simulink model "MX_Gateway"  (Simulink 9.0) (continued)
== stateflow chart MX_Gtwy_Control/System/EMEA Control Mode/Control Modes ==
  state Non_Functional
    en:
    mode_LeftControl = MODE_LEFT_OFFSET;
    mode_RightControl = MODE_RIGHT_AUX1;
    check_LeftNeutral = 0;
    check_RightNeutral = 0;
    flag_AuxEnableFlash = 0;
    aux_inhibit = 0;
    display_request = 0;
    stored_ControlMode = EE_NA_NA;
  state OptionA
    state Left_Control_or_Diverter_Fault
      en:
      flag_AuxEnableFlash = 0;
      check_LeftNeutral = 0;
      state Mode_None_Aux1
        en:
        mode_LeftControl = MODE_LEFT_NONE;
        mode_RightControl = MODE_RIGHT_AUX1;
      state Fault_Mode
        en:
        mode_LeftControl = MODE_LEFT_NONE;
        mode_RightControl = MODE_RIGHT_NONE;
        stored_ControlMode = EE_NA_NA;
      (default) -> Fault_Mode
      Fault_Mode -> Mode_None_Aux1 : [state_AuxEnable != INACTIVE]
      Mode_None_Aux1 -> Fault_Mode : [state_AuxEnable == INACTIVE]
    state INIT_SystemCheck
      en:
      mode_LeftControl = MODE_LEFT_NONE;
      mode_RightControl = MODE_RIGHT_NONE;
      display_request = 0;
      flag_AuxEnableFlash = 0;
      check_LeftNeutral = 0;
    state Delay
    note: Need brief delay here to make sure state_AuxEnable gets set
        | from aux enable model
    state Enabled
      state Aux_Enabled
        en:
        flag_AuxEnableFlash = 0;
        state Mode_Aux2_Aux1
          en:
          mode_LeftControl = MODE_LEFT_AUX2;
          mode_RightControl = MODE_RIGHT_AUX1;
          stored_ControlMode = EE_LT_A2_A1;
          du:
          check_LeftNeutral = 0;
        state Mode_Offset_Aux1
          en:
          mode_LeftControl = MODE_LEFT_OFFSET;
          mode_RightControl = MODE_RIGHT_AUX1;
          stored_ControlMode = EE_LT_OF_A1;
          display_request = 0;
          du:
          check_LeftNeutral = 0;
        (default) -> OptionA.Enabled.Aux_Enabled.Mode_Aux2_Aux1 [order 1] : [stored_ControlMode == EE_LT_A2_A1]
        (default) -> OptionA.Enabled.Aux_Enabled.Mode_Offset_Aux1 [order 2]
        OptionA.Enabled.Aux_Enabled.Mode_Offset_Aux1 -> (junction 5996) : [change_LeftControlMode]
            | {check_LeftNeutral = 1; }
        (junction 5996) -> OptionA.Enabled.Aux_Enabled.Mode_Aux2_Aux1 [order 1] : [valid_LeftControl]
            | {state_Buzzer = DOUBLE_SHORT_BEEP; }
        (junction 5996) -> OptionA.Enabled.Aux_Enabled.Mode_Offset_Aux1 [order 2]
        (junction 5997) -> OptionA.Enabled.Aux_Enabled.Mode_Offset_Aux1 [order 1] : [valid_LeftControl]
            | {state_Buzzer = SINGLE_SHORT_BEEP;}
        OptionA.Enabled.Aux_Enabled.Mode_Aux2_Aux1 -> (junction 5997) : [change_LeftControlMode]
            | {check_LeftNeutral = 1; }
        (junction 5997) -> OptionA.Enabled.Aux_Enabled.Mode_Aux2_Aux1 [order 2]
      state Mode_Offset_None
        en:
        mode_LeftControl = MODE_LEFT_OFFSET;
        mode_RightControl = MODE_RIGHT_NONE;
        stored_ControlMode = EE_LT_OF_NA;
        du:
        check_LeftNeutral = 0;
        on after(100, msec):
        display_request = 0;
        on after(2000, msec):
        flag_AuxEnableFlash = 0;
      (default) -> OptionA.Enabled.Mode_Offset_None [order 2]
      (default) -> OptionA.Enabled.Aux_Enabled [order 1] : [state_AuxEnable != INACTIVE]
      OptionA.Enabled.Mode_Offset_None -> OptionA.Enabled.Aux_Enabled [order 1] : [state_AuxEnable != INACTIVE]
      OptionA.Enabled.Aux_Enabled -> OptionA.Enabled.Mode_Offset_None [order 1] : [state_AuxEnable == INACTIVE]
      OptionA.Enabled.Mode_Offset_None -> (junction 5987) [order 2] : [change_LeftControlMode]
      (junction 5987) -> (junction 5988) : {state_Buzzer = TRIPLE_SHORT_BEEP;
          |  display_request = 1;
          |   flag_AuxEnableFlash = 1;}
      (junction 5988) -> OptionA.Enabled.Mode_Offset_None
    (default) -> OptionA.INIT_SystemCheck
    Left_Control_or_Diverter_Fault -> OptionA.INIT_SystemCheck : [state_Console != ACTIVE...
        | || state_System != SYS_NORMAL...
        | || state_Engine == ENGINE_CRANKING]
    OptionA.Enabled -> OptionA.INIT_SystemCheck : [state_Console != ACTIVE...
        | || state_System != SYS_NORMAL...
        | || state_Engine == ENGINE_CRANKING]
    OptionA.INIT_SystemCheck -> (junction 5975) : [state_Console == ACTIVE...
        | && state_Engine != ENGINE_CRANKING...
        | && state_System == SYS_NORMAL...
        | && flag_InitEERead]
    (junction 5975) -> Left_Control_or_Diverter_Fault [order 2]
    (junction 5975) -> OptionA.Delay [order 1] : [valid_LeftControl]
    OptionA.Enabled.Aux_Enabled -> Left_Control_or_Diverter_Fault [order 2] : [fault_Diverter]
    OptionA.Delay -> OptionA.Enabled
  state OptionB
    state INIT_SystemCheck
      en:
      mode_LeftControl = MODE_LEFT_NONE;
      mode_RightControl = MODE_RIGHT_NONE;
      flag_AuxEnableFlash = 0;
      aux_inhibit = 0;
      display_request = 0;
      check_LeftNeutral = 0;
      check_RightNeutral = 0;
    note: NOTES:
        | cal_RightControl and cal_LeftControl are used to trigger a parallel pot neutral check & and offset adjustment over in the control input models. Must keep the neutral checking limits the same in both models.
        | 2) if pot out of neutral occurs when switching offset, latch into a fault state where nothing works, only key cycle or switching offset back with recover. Aux enable must also be non-functional in this state.
        | 3) must look for a change in state when checking pot on aux enable going active so system doesnt just enable if pot returns to neutral. Aux enable must also be non-functional during this condition
        | 4) if stored offset is on opposite side, disable aux and go to default state
    note: Need a delay here to make sure we received PGN FF35
        | CAN01 is used for state_Comm, and FF35 may come later
    state Delay
    note: If no comm at startup, assume offset left operation
    state Enabled
      state Offset_Left
        state Aux_Enabled
          en:
          flag_AuxEnableFlash = 0;
          state Mode_Offset_Aux4
            en:
            mode_LeftControl = MODE_LEFT_OFFSET;
            mode_RightControl = MODE_RIGHT_AUX4;
            ee_ControlMode = EE_LT_OF_A4;
            matlab_UpdateEEVar();
            du:
            check_LeftNeutral = 0;
            check_RightNeutral = 0;
          state Mode_Offset_Aux1
            en:
            mode_LeftControl = MODE_LEFT_OFFSET;
            mode_RightControl = MODE_RIGHT_AUX1;
            flag_AuxEnableFlash = 0;
            ee_ControlMode = EE_LT_OF_A1;
            matlab_UpdateEEVar();
            du:
            check_LeftNeutral = 0;
            check_RightNeutral = 0;
          (default) -> OptionB.Enabled.Offset_Left.Aux_Enabled.Mode_Offset_Aux4 [order 1] : [ee_ControlMode == EE_LT_OF_A4]
          (default) -> OptionB.Enabled.Offset_Left.Aux_Enabled.Mode_Offset_Aux1 [order 2]
          OptionB.Enabled.Offset_Left.Aux_Enabled.Mode_Offset_Aux1 -> (junction 6067) : [change_RightControlMode...
              | && state_CommSecondary == NORMAL]
              | {check_RightNeutral = 1; }
          (junction 6067) -> OptionB.Enabled.Offset_Left.Aux_Enabled.Mode_Offset_Aux4 [order 1] : [valid_RightControl]
              | {state_Buzzer = DOUBLE_SHORT_BEEP;}
          (junction 6067) -> OptionB.Enabled.Offset_Left.Aux_Enabled.Mode_Offset_Aux1 [order 2]
          (junction 6068) -> OptionB.Enabled.Offset_Left.Aux_Enabled.Mode_Offset_Aux1 [order 1] : [valid_RightControl]
              | {state_Buzzer = SINGLE_SHORT_BEEP; }
          OptionB.Enabled.Offset_Left.Aux_Enabled.Mode_Offset_Aux4 -> (junction 6068) [order 1] : [change_RightControlMode]
              | {check_RightNeutral = 1; }
          (junction 6068) -> OptionB.Enabled.Offset_Left.Aux_Enabled.Mode_Offset_Aux4 [order 2]
        state Mode_Offset_None
          en:
          mode_LeftControl = MODE_LEFT_OFFSET;
          mode_RightControl = MODE_RIGHT_NONE;
          du:
          check_LeftNeutral = 0;
          check_RightNeutral = 0;
          aux_inhibit = 0;
          on after(100, msec):
          display_request = 0;
          on after(2000, msec):
          flag_AuxEnableFlash = 0;
        (default) -> (junction 6057) [order 1] : [state_AuxEnable != INACTIVE]
        (default) -> OptionB.Enabled.Offset_Left.Mode_Offset_None [order 2]
        (junction 6057) -> OptionB.Enabled.Offset_Left.Aux_Enabled [order 1] : [ee_ControlMode < EE_RT_NA_OF]
        (junction 6057) -> (junction 6058) [order 2] : {aux_inhibit = 1;}
            | %Note 4
        OptionB.Enabled.Offset_Left.Mode_Offset_None -> OptionB.Enabled.Offset_Left.Mode_Offset_None [order 1] : [change_RightControlMode]
            | {state_Buzzer = TRIPLE_SHORT_BEEP;
            |  display_request = 1;
            | flag_AuxEnableFlash = 1;}
        (junction 6058) -> OptionB.Enabled.Offset_Left.Mode_Offset_None
        OptionB.Enabled.Offset_Left.Mode_Offset_None -> OptionB.Enabled.Offset_Left.Aux_Enabled [order 2] : [state_AuxEnable != INACTIVE]
        OptionB.Enabled.Offset_Left.Aux_Enabled -> OptionB.Enabled.Offset_Left.Mode_Offset_None : [state_AuxEnable == INACTIVE]
            | {ee_ControlMode = EE_NA_NA;
            | matlab_UpdateEEVar();}
      state Control_Fault
        en:
        mode_LeftControl = MODE_LEFT_NONE;
        mode_RightControl = MODE_RIGHT_NONE;
        aux_inhibit = 1;
        flag_AuxEnableFlash = 0;
        ee_ControlMode = EE_NA_NA;
        matlab_UpdateEEVar();
        du:
        check_LeftNeutral = 0;
        check_RightNeutral = 0;
        ex:
        aux_inhibit = 0;
        %Note 2
      state Offset_Right
        state Aux_Enabled
          en:
          flag_AuxEnableFlash = 0;
          state Mode_Aux4_Aux1
            en:
            mode_LeftControl = MODE_LEFT_AUX4;
            mode_RightControl = MODE_RIGHT_AUX1;
            ee_ControlMode = EE_RT_A4_A1;
            matlab_UpdateEEVar();
            du:
            check_LeftNeutral = 0;
            check_RightNeutral = 0;
          state Mode_Aux4_Offset
            en:
            mode_LeftControl = MODE_LEFT_AUX4;
            mode_RightControl = MODE_RIGHT_OFFSET;
            flag_AuxEnableFlash = 0;
            ee_ControlMode = EE_RT_A4_OF;
            matlab_UpdateEEVar();
            du:
            check_LeftNeutral = 0;
            check_RightNeutral = 0;
            on after(100, msec):
            display_request = 0;
          (default) -> OptionB.Enabled.Offset_Right.Aux_Enabled.Mode_Aux4_Offset [order 2]
          (default) -> OptionB.Enabled.Offset_Right.Aux_Enabled.Mode_Aux4_Aux1 [order 1] : [ee_ControlMode == EE_RT_A4_A1]
          OptionB.Enabled.Offset_Right.Aux_Enabled.Mode_Aux4_Offset -> (junction 6090) : [change_RightControlMode]
              | {check_RightNeutral = 1; }
          (junction 6090) -> OptionB.Enabled.Offset_Right.Aux_Enabled.Mode_Aux4_Aux1 [order 1] : [valid_RightControl]
              | {state_Buzzer = DOUBLE_SHORT_BEEP;}
          (junction 6090) -> OptionB.Enabled.Offset_Right.Aux_Enabled.Mode_Aux4_Offset [order 2]
          (junction 6091) -> OptionB.Enabled.Offset_Right.Aux_Enabled.Mode_Aux4_Offset [order 1] : [valid_RightControl]
              | {state_Buzzer = SINGLE_SHORT_BEEP; }
          OptionB.Enabled.Offset_Right.Aux_Enabled.Mode_Aux4_Aux1 -> (junction 6091) : [change_RightControlMode]
              | {check_RightNeutral = 1; }
          (junction 6091) -> OptionB.Enabled.Offset_Right.Aux_Enabled.Mode_Aux4_Aux1 [order 2]
        state Mode_None_Offset
          en:
          mode_LeftControl = MODE_LEFT_NONE;
          mode_RightControl = MODE_RIGHT_OFFSET;
          du:
          check_LeftNeutral = 0;
          check_RightNeutral = 0;
          aux_inhibit = 0;
          on after(100, msec):
          display_request = 0;
          on after(2000, msec):
          flag_AuxEnableFlash = 0;
        (default) -> (junction 6077) [order 1] : [state_AuxEnable != INACTIVE]
        (default) -> OptionB.Enabled.Offset_Right.Mode_None_Offset [order 2]
        (junction 6077) -> OptionB.Enabled.Offset_Right.Aux_Enabled [order 1] : [ee_ControlMode >= EE_RT_NA_OF]
        (junction 6077) -> (junction 6078) [order 2] : {aux_inhibit = 1;}
            | %Note 4
        (junction 6078) -> OptionB.Enabled.Offset_Right.Mode_None_Offset
        OptionB.Enabled.Offset_Right.Mode_None_Offset -> (junction 6079) [order 2] : [change_AuxEnable...
            | && state_AuxEnable != INACTIVE...
            | && state_CommSecondary == NORMAL]
            | {check_LeftNeutral = 1;}
            | %Note 3
        (junction 6079) -> OptionB.Enabled.Offset_Right.Aux_Enabled [order 1] : [valid_LeftControl]
        (junction 6079) -> OptionB.Enabled.Offset_Right.Mode_None_Offset [order 2] : {aux_inhibit = 1;}
        OptionB.Enabled.Offset_Right.Mode_None_Offset -> OptionB.Enabled.Offset_Right.Mode_None_Offset [order 1] : [change_RightControlMode]
            | {state_Buzzer = TRIPLE_SHORT_BEEP;
            |  display_request = 1;
            | flag_AuxEnableFlash = 1;}
        OptionB.Enabled.Offset_Right.Aux_Enabled -> OptionB.Enabled.Offset_Right.Mode_None_Offset [order 1] : [state_AuxEnable == INACTIVE]
            | {ee_ControlMode = EE_NA_NA;
            | matlab_UpdateEEVar();}
      OptionB.Enabled.Offset_Left.Aux_Enabled.Mode_Offset_Aux4 -> (junction 6040) [order 2] : [state_CommSecondary == NO_COMMUNICATION]
      OptionB.Enabled.Offset_Left -> (junction 6037) : [change_OffsetSelectSw...
          | && din_OffsetSelectSw == OFFSET_SELECT_RIGHT...
          | && state_CommSecondary == NORMAL]
          | {check_RightNeutral = 1;}
      (junction 6038) -> OptionB.Enabled.Offset_Left [order 1] : [valid_LeftControl]
          | {state_Buzzer = SINGLE_MEDIUM_BEEP;
          | flag_AuxEnableFlash = 1; }
      OptionB.Enabled.Control_Fault -> (junction 6037) [order 1] : [change_OffsetSelectSw...
          | && din_OffsetSelectSw == OFFSET_SELECT_RIGHT...
          | && state_CommSecondary == NORMAL]
          | {check_RightNeutral = 1;}
      (junction 6038) -> OptionB.Enabled.Control_Fault [order 2]
      (junction 6037) -> (junction 6041)
      (junction 6042) -> (junction 6038)
      (junction 6039) -> OptionB.Enabled.Control_Fault
      (junction 6040) -> (junction 6039)
      OptionB.Enabled.Offset_Right.Aux_Enabled -> (junction 6040) [order 2] : [state_CommSecondary == NO_COMMUNICATION]
      (junction 6041) -> OptionB.Enabled.Control_Fault [order 2]
      OptionB.Enabled.Control_Fault -> (junction 6042) [order 2] : [change_OffsetSelectSw...
          | && din_OffsetSelectSw == OFFSET_SELECT_LEFT...
          | && state_CommSecondary == NORMAL]
          | {check_LeftNeutral = 1; }
      (junction 6041) -> OptionB.Enabled.Offset_Right [order 1] : [valid_RightControl]
          | {state_Buzzer = SINGLE_MEDIUM_BEEP;
          | flag_AuxEnableFlash = 1;}
      OptionB.Enabled.Offset_Right -> (junction 6042) : [change_OffsetSelectSw...
          | && din_OffsetSelectSw == OFFSET_SELECT_LEFT]
          | {check_LeftNeutral = 1; }
    (default) -> OptionB.INIT_SystemCheck
    OptionB.INIT_SystemCheck -> (junction 6006) : [state_Console == ACTIVE...
        | && state_System == SYS_NORMAL...
        | && state_Engine != ENGINE_CRANKING...
        | && flag_InitEERead]
    OptionB.Enabled -> OptionB.INIT_SystemCheck : [state_Console != ACTIVE...
        | || state_System != SYS_NORMAL...
        | || state_Engine == ENGINE_CRANKING]
    (junction 6006) -> OptionB.Delay [order 1] : [state_CommSecondary == NORMAL]
    (junction 6006) -> (junction 6010) [order 2]
    OptionB.Delay -> (junction 6009) [order 1] : [after(200, msec)]
    OptionB.Delay -> (junction 6014) [order 2] : [state_CommSecondary == NO_COMMUNICATION]
    (junction 6008) -> (junction 6007) [order 1] : [valid_RightControl]
    (junction 6009) -> (junction 6008) [order 1] : [din_OffsetSelectSw == OFFSET_SELECT_RIGHT]
    (junction 6007) -> (junction 6016)
    (junction 6008) -> (junction 6012) [order 2] : {check_RightNeutral = 1;}
    (junction 6009) -> (junction 6014) [order 2]
    (junction 6010) -> (junction 6014) : [state_CommSecondary == NO_COMMUNICATION]
    (junction 6012) -> (junction 6011)
    (junction 6013) -> (junction 6012) [order 2] : {check_LeftNeutral = 1; }
    (junction 6014) -> (junction 6013) : /*[din_OffsetSelectSw == OFFSET_SELECT_LEFT]*/
    (junction 6011) -> (junction 6015)
    (junction 6013) -> OptionB.Enabled.Offset_Left [order 1] : [valid_LeftControl]
    (junction 6015) -> OptionB.Enabled.Control_Fault
    (junction 6016) -> OptionB.Enabled.Offset_Right
  state OptionC
    state INIT_SystemCheck
      en:
      mode_LeftControl = MODE_LEFT_NONE;
      mode_RightControl = MODE_RIGHT_NONE;
      flag_AuxEnableFlash = 0;
      aux_inhibit = 0;
      display_request = 0;
      check_LeftNeutral = 0;
      check_RightNeutral = 0;
    note: NOTES:
        | cal_RightControl and cal_LeftControl are used to trigger a parallel pot neutral check & and offset adjustment over in the control input models. Must keep the neutral checking limits the same in both models.
        | 2) if pot out of neutral occurs when switching offset, latch into a fault state where nothing works, only key cycle or switching offset back with recover. Aux enable must also be non-functional in this state.
        | 3) must look for a change in state when checking pot on aux enable going active so system doesnt just enable if pot returns to neutral. Aux enable must also be non-functional during this condition
        | 4) if stored offset is on opposite side, disable aux and go to default state
    note: Need a delay here to make sure we received PGN FF35
        | CAN01 is used for state_Comm, and FF35 may come later
    state Delay
    state DiverterFaultOverrideLeft [function]
      note: Since diverter related outputs (offset/Aux2) are disabled
          | during a diverter fault - overwrite the mode to none
      (default) -> (junction 6131)
      (junction 6131) -> (junction 6132) [order 1] : [fault_Diverter]
      (junction 6131) -> (junction 6133) [order 2]
      (junction 6132) -> (junction 6134) : {
          | mode_LeftControl = MODE_LEFT_NONE;
          | }
      (junction 6134) -> (junction 6133)
      (junction 6133) -> (junction 6135)
    state DiverterFaultOverrideRight [function]
      note: Since diverter related outputs (offset/Aux2) are disabled
          | during a diverter fault - overwrite the mode to none
      (default) -> (junction 6142)
      (junction 6142) -> (junction 6143) [order 1] : [fault_Diverter]
      (junction 6142) -> (junction 6144) [order 2]
      (junction 6143) -> (junction 6145) : {
          | mode_RightControl = MODE_RIGHT_NONE;
          | }
      (junction 6145) -> (junction 6144)
      (junction 6144) -> (junction 6146)
    note: If no comm at startup, assume offset left operation
    state Enabled
      state Offset_Left
        state Aux_Enabled
          en:
          flag_AuxEnableFlash = 0;
          state Mode_Offset_Aux4
            en:
            mode_LeftControl = MODE_LEFT_OFFSET;
            mode_RightControl = MODE_RIGHT_AUX4;
            ee_ControlMode = EE_LT_OF_A4;
            matlab_UpdateEEVar();
            DiverterFaultOverrideLeft();
            du:
            check_LeftNeutral = 0;
            check_RightNeutral = 0;
            DiverterFaultOverrideLeft();
          state Mode_Offset_Aux1
            en:
            mode_LeftControl = MODE_LEFT_OFFSET;
            mode_RightControl = MODE_RIGHT_AUX1;
            ee_ControlMode = EE_LT_OF_A1;
            matlab_UpdateEEVar();
            DiverterFaultOverrideLeft();
            du:
            check_LeftNeutral = 0;
            check_RightNeutral = 0;
            DiverterFaultOverrideLeft();
          state Mode_Aux2_Aux4
            en:
            mode_LeftControl = MODE_LEFT_AUX2;
            mode_RightControl = MODE_RIGHT_AUX4;
            ee_ControlMode = EE_LT_A2_A4;
            matlab_UpdateEEVar();
            DiverterFaultOverrideLeft();
            du:
            check_LeftNeutral = 0;
            check_RightNeutral = 0;
            DiverterFaultOverrideLeft();
          state Mode_Aux2_Aux1
            en:
            mode_LeftControl = MODE_LEFT_AUX2;
            mode_RightControl = MODE_RIGHT_AUX1;
            ee_ControlMode = EE_LT_A2_A1;
            matlab_UpdateEEVar();
            DiverterFaultOverrideLeft();
            du:
            check_LeftNeutral = 0;
            check_RightNeutral = 0;
            DiverterFaultOverrideLeft();
          (default) -> OptionC.Enabled.Offset_Left.Aux_Enabled.Mode_Offset_Aux4 [order 1] : [ee_ControlMode == EE_LT_OF_A4]
          (default) -> OptionC.Enabled.Offset_Left.Aux_Enabled.Mode_Offset_Aux1 [order 4]
          OptionC.Enabled.Offset_Left.Aux_Enabled.Mode_Offset_Aux1 -> (junction 6186) [order 2] : [change_RightControlMode...
              | && state_CommSecondary == NORMAL]
              | {check_RightNeutral = 1; }
          (junction 6186) -> OptionC.Enabled.Offset_Left.Aux_Enabled.Mode_Offset_Aux4 [order 1] : [valid_RightControl]
              | {state_Buzzer = DOUBLE_SHORT_BEEP;}
          (junction 6186) -> OptionC.Enabled.Offset_Left.Aux_Enabled.Mode_Offset_Aux1 [order 2]
          OptionC.Enabled.Offset_Left.Aux_Enabled.Mode_Offset_Aux4 -> (junction 6187) [order 3] : [state_CommSecondary == NO_COMMUNICATION]
          (junction 6188) -> OptionC.Enabled.Offset_Left.Aux_Enabled.Mode_Offset_Aux1 [order 1] : [valid_RightControl]
              | {state_Buzzer = SINGLE_SHORT_BEEP; }
          OptionC.Enabled.Offset_Left.Aux_Enabled.Mode_Offset_Aux4 -> (junction 6188) [order 1] : [change_RightControlMode]
              | {check_RightNeutral = 1; }
          (junction 6188) -> OptionC.Enabled.Offset_Left.Aux_Enabled.Mode_Offset_Aux4 [order 2]
          (junction 6190) -> OptionC.Enabled.Offset_Left.Aux_Enabled.Mode_Offset_Aux4 [order 2]
          OptionC.Enabled.Offset_Left.Aux_Enabled.Mode_Offset_Aux4 -> (junction 6190) [order 2] : [change_LeftControlMode...
              | && !fault_Diverter]
              | {check_LeftNeutral = 1; }
          (junction 6191) -> OptionC.Enabled.Offset_Left.Aux_Enabled.Mode_Offset_Aux4 [order 1] : [valid_LeftControl]
              | {state_Buzzer = SINGLE_SHORT_BEEP; }
          (junction 6192) -> OptionC.Enabled.Offset_Left.Aux_Enabled.Mode_Offset_Aux1 [order 2]
          OptionC.Enabled.Offset_Left.Aux_Enabled.Mode_Offset_Aux1 -> (junction 6192) [order 1] : [change_LeftControlMode...
              | && !fault_Diverter]
              | {check_LeftNeutral = 1; }
          (junction 6189) -> OptionC.Enabled.Offset_Left.Aux_Enabled.Mode_Offset_Aux1 [order 1] : [valid_LeftControl]
              | {state_Buzzer = SINGLE_SHORT_BEEP; }
          (junction 6189) -> OptionC.Enabled.Offset_Left.Aux_Enabled.Mode_Aux2_Aux1 [order 2]
          (junction 6191) -> Mode_Aux2_Aux4 [order 2]
          OptionC.Enabled.Offset_Left.Aux_Enabled.Mode_Aux2_Aux1 -> (junction 6189) [order 2] : [change_LeftControlMode...
              | && !fault_Diverter]
              | {check_LeftNeutral = 1; }
          (junction 6190) -> Mode_Aux2_Aux4 [order 1] : [valid_LeftControl]
              | {state_Buzzer = DOUBLE_SHORT_BEEP; }
          Mode_Aux2_Aux4 -> (junction 6191) [order 1] : [change_LeftControlMode...
              | && !fault_Diverter]
              | {check_LeftNeutral = 1; }
          (junction 6192) -> OptionC.Enabled.Offset_Left.Aux_Enabled.Mode_Aux2_Aux1 [order 1] : [valid_LeftControl]
              | {state_Buzzer = DOUBLE_SHORT_BEEP; }
          OptionC.Enabled.Offset_Left.Aux_Enabled.Mode_Aux2_Aux1 -> (junction 6193) [order 1] : [change_RightControlMode...
              | && state_CommSecondary == NORMAL]
              | {check_RightNeutral = 1; }
          (junction 6193) -> Mode_Aux2_Aux4 [order 1] : [valid_RightControl]
              | {state_Buzzer = DOUBLE_SHORT_BEEP;}
          (junction 6193) -> OptionC.Enabled.Offset_Left.Aux_Enabled.Mode_Aux2_Aux1 [order 2]
          (default) -> OptionC.Enabled.Offset_Left.Aux_Enabled.Mode_Aux2_Aux1 [order 2] : [ee_ControlMode == EE_LT_A2_A1]
          (default) -> Mode_Aux2_Aux4 [order 3] : [ee_ControlMode == EE_LT_A2_A4]
          (junction 6194) -> OptionC.Enabled.Offset_Left.Aux_Enabled.Mode_Aux2_Aux1 [order 1] : [valid_RightControl]
              | {state_Buzzer = SINGLE_SHORT_BEEP; }
          Mode_Aux2_Aux4 -> (junction 6194) [order 2] : [change_RightControlMode]
              | {check_RightNeutral = 1; }
          (junction 6194) -> Mode_Aux2_Aux4 [order 2]
        state Mode_Offset_None
          en:
          mode_LeftControl = MODE_LEFT_OFFSET;
          mode_RightControl = MODE_RIGHT_NONE;
          DiverterFaultOverrideLeft();
          du:
          check_LeftNeutral = 0;
          check_RightNeutral = 0;
          aux_inhibit = 0;
          DiverterFaultOverrideLeft();
          on after(100, msec):
          display_request = 0;
          on after(2000, msec):
          flag_AuxEnableFlash = 0;
        (default) -> (junction 6176) [order 1] : [state_AuxEnable != INACTIVE]
        (default) -> OptionC.Enabled.Offset_Left.Mode_Offset_None [order 2]
        (junction 6176) -> OptionC.Enabled.Offset_Left.Aux_Enabled [order 1] : [ee_ControlMode < EE_RT_NA_OF]
        (junction 6176) -> (junction 6177) [order 2] : {aux_inhibit = 1;}
            | %Note 4
        OptionC.Enabled.Offset_Left.Mode_Offset_None -> OptionC.Enabled.Offset_Left.Mode_Offset_None [order 1] : [change_LeftControlMode...
            | || change_RightControlMode]
            | {state_Buzzer = TRIPLE_SHORT_BEEP;
            | display_request = 1;
            | flag_AuxEnableFlash = 1;}
        (junction 6177) -> OptionC.Enabled.Offset_Left.Mode_Offset_None
        OptionC.Enabled.Offset_Left.Mode_Offset_None -> OptionC.Enabled.Offset_Left.Aux_Enabled [order 2] : [state_AuxEnable != INACTIVE]
        OptionC.Enabled.Offset_Left.Aux_Enabled -> OptionC.Enabled.Offset_Left.Mode_Offset_None : [state_AuxEnable == INACTIVE]
            | {ee_ControlMode = EE_NA_NA;
            | matlab_UpdateEEVar();}
      state Control_Fault
        en:
        mode_LeftControl = MODE_LEFT_NONE;
        mode_RightControl = MODE_RIGHT_NONE;
        aux_inhibit = 1;
        flag_AuxEnableFlash = 0;
        ee_ControlMode = EE_NA_NA;
        matlab_UpdateEEVar();
        du:
        check_LeftNeutral = 0;
        check_RightNeutral = 0;
        ex:
        aux_inhibit = 0;
        %Note 2
      state Offset_Right
        state Aux_Enabled
          en:
          flag_AuxEnableFlash = 0;
          state Mode_Aux4_Aux1
            en:
            mode_LeftControl = MODE_LEFT_AUX4;
            mode_RightControl = MODE_RIGHT_AUX1;
            ee_ControlMode = EE_RT_A4_A1;
            matlab_UpdateEEVar();
            du:
            check_LeftNeutral = 0;
            check_RightNeutral = 0;
          state Mode_Aux4_Offset
            en:
            mode_LeftControl = MODE_LEFT_AUX4;
            mode_RightControl = MODE_RIGHT_OFFSET;
            ee_ControlMode = EE_RT_A4_OF;
            matlab_UpdateEEVar();
            DiverterFaultOverrideRight();
            du:
            check_LeftNeutral = 0;
            check_RightNeutral = 0;
            DiverterFaultOverrideRight();
            on after(100, msec):
            display_request = 0;
          state Mode_Aux2_Aux1
            en:
            mode_LeftControl = MODE_LEFT_AUX2;
            mode_RightControl = MODE_RIGHT_AUX1;
            ee_ControlMode = EE_RT_A2_A1;
            matlab_UpdateEEVar();
            DiverterFaultOverrideLeft();
            du:
            check_LeftNeutral = 0;
            DiverterFaultOverrideLeft();
            on after(100, msec):
            display_request = 0;
          (default) -> OptionC.Enabled.Offset_Right.Aux_Enabled.Mode_Aux4_Offset [order 3]
          (default) -> OptionC.Enabled.Offset_Right.Aux_Enabled.Mode_Aux4_Aux1 [order 1] : [ee_ControlMode == EE_RT_A4_A1]
          OptionC.Enabled.Offset_Right.Aux_Enabled.Mode_Aux4_Offset -> (junction 6237) [order 2] : [change_RightControlMode]
              | {check_RightNeutral = 1; }
          (junction 6237) -> OptionC.Enabled.Offset_Right.Aux_Enabled.Mode_Aux4_Aux1 [order 1] : [valid_RightControl]
              | {state_Buzzer = DOUBLE_SHORT_BEEP;}
          (junction 6237) -> OptionC.Enabled.Offset_Right.Aux_Enabled.Mode_Aux4_Offset [order 2]
          (junction 6238) -> OptionC.Enabled.Offset_Right.Aux_Enabled.Mode_Aux4_Offset [order 1] : [valid_RightControl]
              | {state_Buzzer = SINGLE_SHORT_BEEP; }
          OptionC.Enabled.Offset_Right.Aux_Enabled.Mode_Aux4_Aux1 -> (junction 6238) [order 1] : [change_RightControlMode]
              | {check_RightNeutral = 1; }
          (junction 6238) -> OptionC.Enabled.Offset_Right.Aux_Enabled.Mode_Aux4_Aux1 [order 2]
          (junction 6239) -> OptionC.Enabled.Offset_Right.Aux_Enabled.Mode_Aux4_Aux1 [order 2]
          OptionC.Enabled.Offset_Right.Aux_Enabled.Mode_Aux4_Aux1 -> (junction 6239) [order 2] : [change_LeftControlMode...
              | && !fault_Diverter]
              | {check_LeftNeutral = 1; }
          (junction 6240) -> OptionC.Enabled.Offset_Right.Aux_Enabled.Mode_Aux4_Aux1 [order 1] : [valid_LeftControl]
              | {state_Buzzer = SINGLE_SHORT_BEEP; }
          OptionC.Enabled.Offset_Right.Aux_Enabled.Mode_Aux4_Offset -> OptionC.Enabled.Offset_Right.Aux_Enabled.Mode_Aux4_Offset [order 1] : [change_LeftControlMode]
              | {state_Buzzer = TRIPLE_SHORT_BEEP;
              |  display_request = 1;}
          (junction 6239) -> OptionC.Enabled.Offset_Right.Aux_Enabled.Mode_Aux2_Aux1 [order 1] : [valid_LeftControl]
              | {state_Buzzer = DOUBLE_SHORT_BEEP; }
          (junction 6240) -> OptionC.Enabled.Offset_Right.Aux_Enabled.Mode_Aux2_Aux1 [order 2]
          OptionC.Enabled.Offset_Right.Aux_Enabled.Mode_Aux2_Aux1 -> (junction 6240) [order 2] : [change_LeftControlMode]
              | {check_LeftNeutral = 1; }
          OptionC.Enabled.Offset_Right.Aux_Enabled.Mode_Aux2_Aux1 -> OptionC.Enabled.Offset_Right.Aux_Enabled.Mode_Aux2_Aux1 [order 1] : [change_RightControlMode]
              | {state_Buzzer = TRIPLE_SHORT_BEEP;
              |  display_request = 1;}
          (default) -> OptionC.Enabled.Offset_Right.Aux_Enabled.Mode_Aux2_Aux1 [order 2] : [ee_ControlMode == EE_RT_A2_A1]
        state Mode_None_Offset
          en:
          mode_LeftControl = MODE_LEFT_NONE;
          mode_RightControl = MODE_RIGHT_OFFSET;
          DiverterFaultOverrideRight();
          du:
          check_LeftNeutral = 0;
          check_RightNeutral = 0;
          aux_inhibit = 0;
          DiverterFaultOverrideRight();
          on after(100, msec):
          display_request = 0;
          on after(2000, msec):
          flag_AuxEnableFlash = 0;
        (default) -> (junction 6224) [order 1] : [state_AuxEnable != INACTIVE]
        (default) -> OptionC.Enabled.Offset_Right.Mode_None_Offset [order 2]
        (junction 6224) -> OptionC.Enabled.Offset_Right.Aux_Enabled [order 1] : [ee_ControlMode >= EE_RT_NA_OF]
        (junction 6224) -> (junction 6225) [order 2] : {aux_inhibit = 1;}
            | %Note 4
        (junction 6225) -> OptionC.Enabled.Offset_Right.Mode_None_Offset
        OptionC.Enabled.Offset_Right.Mode_None_Offset -> (junction 6226) [order 2] : [change_AuxEnable...
            | && state_AuxEnable != INACTIVE...
            | && state_CommSecondary == NORMAL]
            | {check_LeftNeutral = 1;}
            | %Note 3
        (junction 6226) -> OptionC.Enabled.Offset_Right.Aux_Enabled [order 1] : [valid_LeftControl]
        (junction 6226) -> OptionC.Enabled.Offset_Right.Mode_None_Offset [order 2] : {aux_inhibit = 1;}
        OptionC.Enabled.Offset_Right.Mode_None_Offset -> OptionC.Enabled.Offset_Right.Mode_None_Offset [order 1] : [change_LeftControlMode...
            | || change_RightControlMode]
            | {state_Buzzer = TRIPLE_SHORT_BEEP;
            |  display_request = 1;
            | flag_AuxEnableFlash = 1;}
        OptionC.Enabled.Offset_Right.Aux_Enabled -> OptionC.Enabled.Offset_Right.Mode_None_Offset [order 1] : [state_AuxEnable == INACTIVE]
            | {ee_ControlMode = EE_NA_NA ;
            | matlab_UpdateEEVar();}
      (junction 6187) -> (junction 6156)
      Mode_Aux2_Aux4 -> (junction 6157) [order 3] : [state_CommSecondary == NO_COMMUNICATION]
      OptionC.Enabled.Offset_Left -> (junction 6153) : [change_OffsetSelectSw...
          | && din_OffsetSelectSw == OFFSET_SELECT_RIGHT...
          | && state_CommSecondary == NORMAL]
          | {check_RightNeutral = 1;}
      (junction 6154) -> OptionC.Enabled.Offset_Left [order 1] : [valid_LeftControl]
          | {state_Buzzer = SINGLE_MEDIUM_BEEP;
          | flag_AuxEnableFlash = 1; }
      OptionC.Enabled.Control_Fault -> (junction 6153) [order 1] : [change_OffsetSelectSw...
          | && din_OffsetSelectSw == OFFSET_SELECT_RIGHT...
          | && state_CommSecondary == NORMAL]
          | {check_RightNeutral = 1;}
      (junction 6154) -> OptionC.Enabled.Control_Fault [order 2]
      (junction 6153) -> (junction 6158)
      (junction 6159) -> (junction 6154)
      (junction 6155) -> OptionC.Enabled.Control_Fault
      (junction 6157) -> (junction 6155)
      (junction 6156) -> (junction 6157)
      OptionC.Enabled.Offset_Right.Aux_Enabled -> (junction 6157) [order 2] : [state_CommSecondary == NO_COMMUNICATION]
      (junction 6158) -> OptionC.Enabled.Control_Fault [order 2]
      OptionC.Enabled.Control_Fault -> (junction 6159) [order 2] : [change_OffsetSelectSw...
          | && din_OffsetSelectSw == OFFSET_SELECT_LEFT...
          | && state_CommSecondary == NORMAL]
          | {check_LeftNeutral = 1; }
      (junction 6158) -> OptionC.Enabled.Offset_Right [order 1] : [valid_RightControl]
          | {state_Buzzer = SINGLE_MEDIUM_BEEP;
          | flag_AuxEnableFlash = 1;}
      OptionC.Enabled.Offset_Right -> (junction 6159) : [change_OffsetSelectSw...
          | && din_OffsetSelectSw == OFFSET_SELECT_LEFT]
          | {check_LeftNeutral = 1; }
    (default) -> OptionC.INIT_SystemCheck
    OptionC.INIT_SystemCheck -> (junction 6100) : [state_Console == ACTIVE...
        | && state_System == SYS_NORMAL...
        | && state_Engine != ENGINE_CRANKING...
        | && flag_InitEERead]
    OptionC.Enabled -> OptionC.INIT_SystemCheck : [state_Console != ACTIVE...
        | || state_System != SYS_NORMAL...
        | || state_Engine == ENGINE_CRANKING]
    (junction 6100) -> OptionC.Delay [order 1] : [state_CommSecondary == NORMAL]
    (junction 6100) -> (junction 6104) [order 2]
    OptionC.Delay -> (junction 6103) [order 1] : [after(200, msec)]
    OptionC.Delay -> (junction 6108) [order 2] : [state_CommSecondary == NO_COMMUNICATION]
    (junction 6102) -> (junction 6101) [order 1] : [valid_RightControl]
    (junction 6103) -> (junction 6102) [order 1] : [din_OffsetSelectSw == OFFSET_SELECT_RIGHT]
    (junction 6101) -> (junction 6110)
    (junction 6102) -> (junction 6106) [order 2] : {check_RightNeutral = 1;}
    (junction 6103) -> (junction 6108) [order 2]
    (junction 6104) -> (junction 6108) : [state_CommSecondary == NO_COMMUNICATION]
    (junction 6106) -> (junction 6105)
    (junction 6107) -> (junction 6106) [order 2] : {check_LeftNeutral = 1; }
    (junction 6108) -> (junction 6107) : /*[din_OffsetSelectSw == OFFSET_SELECT_LEFT]*/
    (junction 6105) -> (junction 6109)
    (junction 6107) -> OptionC.Enabled.Offset_Left [order 1] : [valid_LeftControl]
    (junction 6109) -> OptionC.Enabled.Control_Fault
    (junction 6110) -> OptionC.Enabled.Offset_Right
  (default) -> Non_Functional
  Non_Functional -> OptionA [order 1] : [CD_EMEA_CONTROLS_OPTION_A != NON_FUNCTIONAL]
  Non_Functional -> OptionB [order 2] : [CD_EMEA_CONTROLS_OPTION_B != NON_FUNCTIONAL]
  Non_Functional -> OptionC [order 3] : [CD_EMEA_CONTROLS_OPTION_C != NON_FUNCTIONAL]
  data: flag_AuxEnableFlash output boolean; change_RightControlMode input boolean; state_CommSecondary input uint8; mode_LeftControl output uint8; MODE_LEFT_NONE parameter uint8; change_LeftControlMode input boolean; MODE_LEFT_OFFSET parameter uint8; MODE_LEFT_AUX2 parameter uint8; MODE_LEFT_AUX4 parameter uint8; valid_LeftControl input boolean; valid_RightControl input boolean; state_Buzzer data_store_memory double; DOUBLE_SHORT_BEEP parameter uint8; SINGLE_SHORT_BEEP parameter uint8; NORMAL parameter uint8; CD_EMEA_CONTROLS_OPTION_A parameter uint8; CD_EMEA_CONTROLS_OPTION_B parameter uint8; CD_EMEA_CONTROLS_OPTION_C parameter uint8; NON_FUNCTIONAL parameter uint8; OFFSET_SELECT_LEFT parameter uint8; OFFSET_SELECT_RIGHT parameter uint8; SINGLE_MEDIUM_BEEP parameter uint8; TRIPLE_SHORT_BEEP parameter uint8; mode_RightControl output uint8; INACTIVE parameter uint8; MODE_RIGHT_AUX1 parameter uint8; MODE_RIGHT_AUX4 parameter uint8; MODE_RIGHT_NONE parameter uint8; MODE_RIGHT_OFFSET parameter uint8; state_Console input uint8; state_Engine input uint8; state_System input uint8; flag_InitEERead input boolean; fault_Diverter input boolean; state_AuxEnable input uint8; check_LeftNeutral output boolean; ACTIVE parameter uint8; ENGINE_CRANKING parameter uint8; SYS_NORMAL parameter uint8; check_RightNeutral output boolean; change_AuxEnable input boolean; din_OffsetSelectSw input boolean; change_OffsetSelectSw input boolean; aux_inhibit output boolean; OORH parameter uint8; OORL parameter uint8; NO_COMMUNICATION parameter uint8; display_request output boolean; ee_ControlMode data_store_memory double; EE_RT_A2_A1 parameter uint8; EE_LT_A2_A4 parameter uint8; EE_RT_A4_A1 parameter uint8; EE_RT_A4_OF parameter uint8; EE_NA_NA parameter uint8; EE_RT_NA_OF parameter uint8; EE_LT_OF_A1 parameter uint8; EE_LT_OF_A4 parameter uint8; EE_LT_OF_NA parameter uint8; EE_LT_A2_A1 parameter uint8; stored_ControlMode local uint8
